simulink model "Full_Car"  (R2020b)
settings: Solver=ode23  StartTime=0.0  StopTime=20  RelTol=0.01  AbsTol=0.01
PreLoadFcn:
  | addpath('Functions');
  | addpath('Functions/geometric');
  | addpath('Parameters');
  | vhclParams;
  | Mass = Sprung_mass + Driver_mass + Unsprung_mass_front*2 + Unsprung_mass_rear*2;
  | COG = (Sprung_mass_COG*Sprung_mass + Driver_COG*Driver_mass + Unsprung_mass_COG_front*Unsprung_mass_front*2.*[1 0 1] + Unsprung_mass_COG_rear*Unsprung_mass_rear*2.*[1 0 1])/Mass;
InitFcn:
  | [folder, ~, ~] = fileparts(which (bdroot));
  | cd(folder);
undefined symbols (not defined in the file): aero_CF, aero_CM, aero_pressureCenter, aero_refArea, airDensity, ARB_bcrnk_frontL, ARB_bcrnk_frontR, ARB_bcrnk_rearL, ARB_bcrnk_rearR, ARB_center_front, ARB_center_rear, ARB_damping_front, ARB_damping_rear, ARB_length_front, ARB_length_rear, ARB_pinSlot_correctionFactor_front, ARB_pinSlot_correctionFactor_rear, ARB_stiffness_front, ARB_stiffness_rear, ARB_TieRod_length_front, ARB_TieRod_length_rear, Damper_coefficient_front, Damper_coefficient_rear, Driver_COG, Driver_mass, DriveTrain_brkwyTrq, DriveTrain_brkwyVel, DriveTrain_CoulombTrq, DriveTrain_effcy, DriveTrain_ratio, DriveTrain_viscCoef, Floor_pos, Floor_size, FS_camber, FS_toe, gravity, Grid_size, initialPosition, Rim_mass, RodDiameter, RodThickness, RS_camber, RS_toe, sigbuilder_block, Spring_preload_front, Spring_preload_rear, Spring_stiffness_front, Spring_stiffness_rear, Sprung_mass, Sprung_mass_COG, Tire_mass, Unsprung_mass_COG_front, Unsprung_mass_COG_rear, Unsprung_mass_front, Unsprung_mass_rear, util_generate_grid_x, util_generate_grid_y, util_rodCrossSection, X, X_F ... (+3)
libraries: sm_lib x385, nesl_utility x79, simulink_extras x8, tire x4, fl_ps_legacy_lib x1
(part 2 of 17)

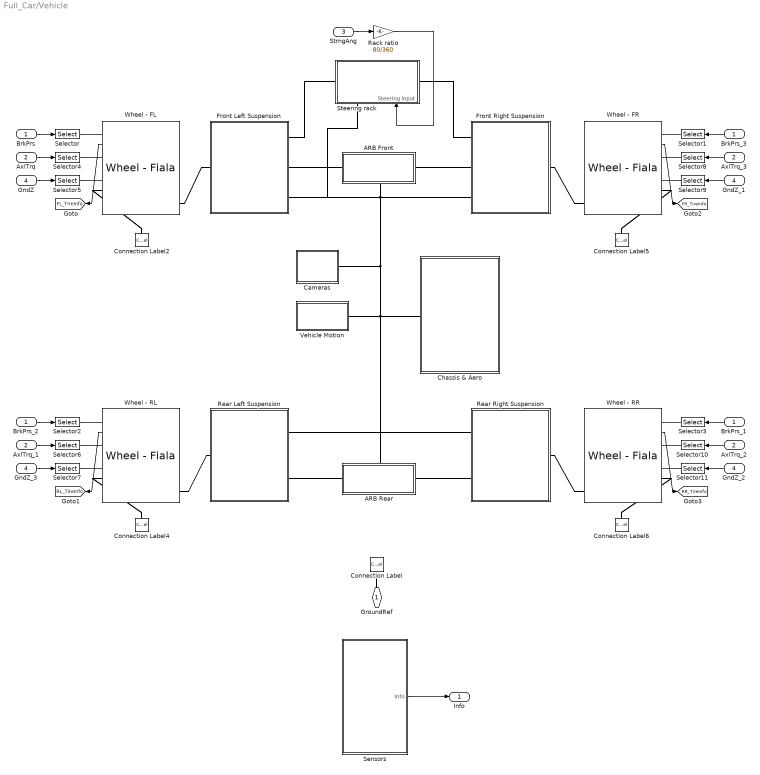
== system Full_Car/Vehicle ==
  BrkPrs : Inport
  AxlTrq : Inport  Port=2
  StrngAng : Inport  Port=3
  GndZ : Inport  Port=4
  ARB Front : Subsystem  mask{arbStiffness=ARB_stiffness_front, arbDamp=ARB_damping_front, arbCorrection=ARB_pinSlot_correctionFactor_front, arbCenter=ARB_center_front, arbLength=ARB_length_front, arbRodLength=ARB_TieRod_length_front, RodDiam=RodDiameter, RodThick=RodThickness}
      mask prompts: arbStiffness "Anti-roll Bar Stiffness"; arbDamp "Anti-roll Bar Damping"; arbCorrection "Stiffness correction factor"; arbCenter "Anti-roll Bar center"; arbLength "Anti-roll Bar length"; arbRodLength "Anti-roll Bar tie-rod length"; RodDiam "Rod diameter"; RodThick "Rod thickness"
      settings: PortSchema="{\"entries\":[{\"content\":{\"sides\":[{\"content\":{\"connectorIds\":[\"LConn1\"]},\"type\":\"ConnectorPlacement.EquallySpacedRectSide\",\"uuid\":\"7b39e7e2-1c99-4477-9d88-8155f0dcbcfa\"},{\"content\":{\"connectorIds\":[\"RConn1\"],\"side\":\"RIGHT\"},\"type\":\"ConnectorPlacement.EquallySpacedRectSide\",\"uuid\":\"e62feb70-34ca-4fb6-b792-0423ade04344\"},{\"content\":{\"connectorIds\":[],\"side\":\"TOP\"},\"type\":\"ConnectorPlacement.EquallySpacedRectSide\",\"uuid\":\"b26fb64d-892c-44a6-8f06-11f439d11cb6\"},{\"content\":{\"connectorIds\":[\"RConn2\"],\"side\":\"BOTTOM\"},\"type\":\"ConnectorPlacement.EquallySpacedRectSide\",\"uuid\":\"e4489596-2281-46d7-9d2e-4ef3bf65c061\"}]},\"type\":\"ConnectorPlacement.EquallySpacedConnector\",\"uuid\":\"4e7c908e-7a44-4075-b019-e55e6c824031\"}],\"packageUris\":[\"http://schema.mathworks.com/mf0/Connector/19700101\"],\"version\":\"1.0\"}"
      mask DialogControl DescGroupVar Prompt: Anti-roll Bar
      mask DialogControl DescTextVar Prompt: Z-Type anti-roll bar assembly
      mask DialogControl ParameterGroupVar Prompt: Dynamics
      mask DialogControl arbStiffness Tooltip: Anti-roll bar stiffness (N/mm)
      mask DialogControl arbDamp Tooltip: Anti-roll bar damping (N/(mm/s))
      mask DialogControl Container4 Prompt: Geometry
      mask DialogControl arbCenter Tooltip: Coordinates of the anti-roll bar center (mm)
      mask DialogControl arbLength Tooltip: Length of the anti-roll bar arm (mm)
      mask DialogControl arbRodLength Tooltip: length of the actuation rod of the anti-roll bar (mm)
      mask DialogControl Container5 Prompt: Graphic
      mask Display: image('$imagefile'); % Use "Edit Image" on toolstrip to change.
  ARB Rear : Subsystem  mask{arbStiffness=ARB_stiffness_rear, arbDamp=ARB_damping_rear, arbCorrection=ARB_pinSlot_correctionFactor_rear, arbCenter=ARB_center_rear, arbLength=ARB_length_rear, arbRodLength=ARB_TieRod_length_rear, RodDiam=RodDiameter, RodThick=RodThickness}
      mask prompts: arbStiffness "Anti-roll Bar Stiffness"; arbDamp "Anti-roll Bar Damping"; arbCorrection "Stiffness correction factor"; arbCenter "Anti-roll Bar center"; arbLength "Anti-roll Bar length"; arbRodLength "Anti-roll Bar tie-rod length"; RodDiam "Rod diameter"; RodThick "Rod thickness"
      settings: PortSchema="{\"entries\":[{\"content\":{\"sides\":[{\"content\":{\"connectorIds\":[\"LConn1\"]},\"type\":\"ConnectorPlacement.EquallySpacedRectSide\",\"uuid\":\"7b39e7e2-1c99-4477-9d88-8155f0dcbcfa\"},{\"content\":{\"connectorIds\":[\"RConn1\"],\"side\":\"RIGHT\"},\"type\":\"ConnectorPlacement.EquallySpacedRectSide\",\"uuid\":\"e62feb70-34ca-4fb6-b792-0423ade04344\"},{\"content\":{\"connectorIds\":[],\"side\":\"TOP\"},\"type\":\"ConnectorPlacement.EquallySpacedRectSide\",\"uuid\":\"b26fb64d-892c-44a6-8f06-11f439d11cb6\"},{\"content\":{\"connectorIds\":[\"RConn2\"],\"side\":\"BOTTOM\"},\"type\":\"ConnectorPlacement.EquallySpacedRectSide\",\"uuid\":\"e4489596-2281-46d7-9d2e-4ef3bf65c061\"}]},\"type\":\"ConnectorPlacement.EquallySpacedConnector\",\"uuid\":\"4e7c908e-7a44-4075-b019-e55e6c824031\"}],\"packageUris\":[\"http://schema.mathworks.com/mf0/Connector/19700101\"],\"version\":\"1.0\"}"
      mask DialogControl DescGroupVar Prompt: Anti-roll Bar
      mask DialogControl DescTextVar Prompt: Z-Type anti-roll bar assembly
      mask DialogControl ParameterGroupVar Prompt: Dynamics
      mask DialogControl arbStiffness Tooltip: Anti-roll bar stiffness (N/mm)
      mask DialogControl arbDamp Tooltip: Anti-roll bar damping (N/(mm/s))
      mask DialogControl Container4 Prompt: Geometry
      mask DialogControl arbCenter Tooltip: Coordinates of the anti-roll bar center (mm)
      mask DialogControl arbLength Tooltip: Length of the anti-roll bar arm (mm)
      mask DialogControl arbRodLength Tooltip: length of the actuation rod of the anti-roll bar (mm)
      mask DialogControl Container5 Prompt: Graphic
      mask Display: image('$imagefile'); % Use "Edit Image" on toolstrip to change.
  AxlTrq_1 : InportShadow  Port=2
  AxlTrq_2 : InportShadow  Port=2
  AxlTrq_3 : InportShadow  Port=2
  BrkPrs_1 : InportShadow
  BrkPrs_2 : InportShadow
  BrkPrs_3 : InportShadow
  Cameras : Subsystem
      mask Display: image('$imagefile'); % Use "Edit Image" on toolstrip to change.
  Chassis & Aero : Subsystem
      settings: PortSchema="{\"entries\":[{\"content\":{\"sides\":[{\"content\":{\"connectorIds\":[]},\"type\":\"ConnectorPlacement.EquallySpacedRectSide\",\"uuid\":\"cbbe6af3-19fb-48b3-8800-b215223b12f6\"},{\"content\":{\"connectorIds\":[],\"side\":\"RIGHT\"},\"type\":\"ConnectorPlacement.EquallySpacedRectSide\",\"uuid\":\"e0ce8cb6-c1e3-4be9-8f24-6f1308fe5f38\"},{\"content\":{\"connectorIds\":[\"LConn1\"],\"side\":\"TOP\"},\"type\":\"ConnectorPlacement.EquallySpacedRectSide\",\"uuid\":\"4e525be5-d309-4877-ab04-513b663981dd\"},{\"content\":{\"connectorIds\":[],\"side\":\"BOTTOM\"},\"type\":\"ConnectorPlacement.EquallySpacedRectSide\",\"uuid\":\"1a8d8adb-4793-4ada-a6b7-ecb65b96140c\"}]},\"type\":\"ConnectorPlacement.EquallySpacedConnector\",\"uuid\":\"0639ed7d-0262-43c4-ade7-52e76f974124\"}],\"packageUris\":[\"http://schema.mathworks.com/mf0/Connector/19700101\"],\"version\":\"1.0\"}"
      mask Display:
      | image('$imagefile'); % Use "Edit Image" on toolstrip to change.
      | port_label('LConn',1,'Ref')
  Connection Label{,2,4..6} (5 alike) : ConnectionLabel  Label=GroundRef
  Front Left Suspension : Subsystem  mask{X=X_F, side=Left, sideSign=1, ARB_bcrnk=ARB_bcrnk_frontL, Camber=FS_camber, Toe=FS_toe, BcrnkPos=0, DamperPos=[0 90 0], ARB_On=on, St_On=on, SpringStiffness=Spring_stiffness_front, SpringPreload=Spring_preload_front, DamperCoef=Damper_coefficient_front, DamperMaxExt=200, DampLimit_MaxK=10000, DampLimit_MaxC=1, DampLimit_MaxTrans=1, DamperMinExt=154, DampLimit_MinK=2000, DampLimit_MinC=1, DampLimit_MinTrans=5, PushSetupLength=0, ToeSetupLength=0, UnsprungMass=Unsprung_mass_front-Tire_mass-Rim_mass, UnsprungMassCOG=Unsprung_mass_COG_front, RodThick=1, RodDiam=14, UCAfore_X=152.984798840791, UCAaft_X=-127.305532779834, UCA_Y=-257.3812, UCA_pushBracket_X=1.13049065291656, UCA_pushBracket_Y=-16.0659716079757, UCA_pushBracket_Z=19.373063236757, LCAfore_X=67.6445283762746, LCAaft_X=-143.178288225724, LCA_Y=-328.86366138683, UCA_Rot=[0.0129952164868874 0.0511310087922121 0.998607402480249;0.0511310087922121 0.997351198186899 -0.0517320733689305;-0.998607402480249 0.0517320733689305 0.0103464146737861], LCA_Rot=[0.000690445618848567 0.0146957287408994 0.99989177356433;0.0146957287408994 0.999783886342046 -0.0147042907877108;-0.99989177356433 0.0147042907877108 0.000474331960894192], BcrnkDamp=69.0006325768104, BcrnkPush=69.0007956041088, BcrnkAng=54.9995482296285, BcrnkRot=[-0.0310893815864683 0.0519417534127774 0.998166070654066;0.0519417534127774 0.997383402646003 -0.0502832216418927;-0.998166070654066 0.0502832216418927 -0.0337059789404655]}
      mask prompts: X "Coordinates"; ARB_bcrnk "ARB tie-rod"; BcrnkPos "Bellcrank initial rotation"; DamperPos "Damper initial position"; ARB_On "Anti-roll bar"; St_On "Steering"; SpringStiffness "Spring stiffness"; SpringPreload "Spring preload"; DamperCoef "Damping Coefficient"; DamperMaxExt "Maximum extension"; DampLimit_MaxK "Max_Stiffness"; DampLimit_MaxC "Max_Damping"; DampLimit_MaxTrans "Max_Transition"; DamperMinExt "Maximum compression"; DampLimit_MinK "Min_Stiffness"; DampLimit_MinC "Min_Damping"; DampLimit_MinTrans "Min_Transition"; PushSetupLength "Push"; ToeSetupLength "Toe"; UnsprungMass "Mass"; UnsprungMassCOG "COG"; RodThick "Rod Thickness"; RodDiam "Rod Diameter"
      mask help: Define a Simscape Multibody model of a double control arm independent suspension
      mask init:
      | dblAarmSusp_init(gcb)
      mask Help:
      | -Geometry:
      |     +Suspension Coordinates: coordinates of the points that define a double wishbone suspension system. The coordinates must always correspond to the LEFT side. The variable (e.g. 'X') containing the data must be a row vector with the following values, in order:
      |       1-A-Arm Upper Front hardpoint. X([1 2 3])
      |       2-A-Arm Upper Rear. X([4 5 6])
      |       3-A-Arm Lower Front. X([7 8 9])
      |       4-A-Arm Lower Rear. X([10 11 12])
      |       5-A-Arm Upper Upright. X([13 14 15])
      |       6-A-Arm Lower Upright. X([16 17 18])
      |       7-Steering Rack. X([19 20 21])
      |       8-Steering Upright. X([22 23 24])
      |       9-Wheel Center. X([25 26 27])
      |       10-Wheel Plane normal vector. X([28 29 30])
      |       11-A-Arm Push Bracket. X([31 32 33])
      |       12-Bellcrank Push Bracket. X([34 35 36])
      |       13-Bellcrank Chassis Bracket. X([37 38 39])
      |       14-Bellcrank Damper Bracket. X([40 41 42])
      |       15-Damper Bracket. X([43 44 45])
      |       16-Bellcrank ARB Bracket. X(46:48)
      |       17-ARB end. X(49:51)
      |       18-ARB center. X(52:54)
      |     +It must be noted that in the model the bellcranks have the same dimensions as defined in the geometry, but the position and orientation is diferent. It is positioned in a vertical plane that contains the a-arm push bracket. The reason is to let the anti-roll bar be symmetric.
      mask TypeOptions side: Left | Right
      mask Callback side:
      | switch get_param(gcb,'side')
      |     case 'Left'
      |         set_param(gcb,'sideSign','1');
      |     case 'Right'
      |         set_param(gcb,'sideSign','-1');
      | end
      mask DialogControl DescGroupVar Prompt: Double Wishbone Suspension
      mask DialogControl SuspGeomTab Prompt: Geometry
      mask DialogControl X Tooltip: Variable containting suspension points coordinates
      mask DialogControl Container22 Prompt: Anti-roll Bar
      mask DialogControl ARB_bcrnk Tooltip: Coordinates of the attachment point of the ARB to the bellcrank (mm)
      mask DialogControl Container8 Prompt: Wheel angles
      mask DialogControl Camber Tooltip: Camber angle in deg at reference ride height
      mask DialogControl Toe Tooltip: Toe angle in deg at reference ride height
      mask DialogControl Container23 Prompt: Assembly
      mask DialogControl BcrnkPos Tooltip: Degrees of rotation of follower frame respect to base frame of the bellcrank bracket joint for the inital position
      mask DialogControl DamperPos Tooltip: Degrees of rotation of follower frame respect to base frame of the bellcrank bracket joint for the inital position, specified as a rotation sequence X-Y-Z
      mask DialogControl ARB_On Tooltip: Select wheter the block displays connection for ARB or not
      mask DialogControl St_On Tooltip: Select wether the block displays steering input or not
      mask DialogControl SpringDampTab Prompt: Spring and Damper
      mask DialogControl SpringStiffness Tooltip: Spring stiffness (lb/inch)
      mask DialogControl SpringPreload Tooltip: Spring preload in mm
      mask DialogControl DamperCoef Tooltip: Damping Coefficient in N/(mm/s)
      mask DialogControl Container9 Prompt: Limits
      mask DialogControl DamperMaxExt Tooltip: Maximum allowable extension of the damper, meassured between ball-joints. This value is also used for the natural length of the spring (mm)
      mask DialogControl DampLimit_MaxK Tooltip: Stiffness when upper limit is reached in N/mm
      mask DialogControl DampLimit_MaxC Tooltip: Damping coefficient when upper limit is reached in N/(mm/s)
      mask DialogControl DampLimit_MaxTrans Tooltip: Transition region width between normal behavior and limit
      mask DialogControl DamperMinExt Tooltip: Minimum allowable extension (max compression) of the damper, meassured between ball-joints (mm)
      mask DialogControl DampLimit_MinK Tooltip: Stiffness when lower limit is reached in N/(mm/s)
      mask DialogControl DampLimit_MinC Tooltip: Damping coefficient when lower limit is reached in N/(mm/s)
      mask DialogControl DampLimit_MinTrans Tooltip: Transition region width between normal behavior and limit
      mask DialogControl Container13 Prompt: Setup
      mask DialogControl PushSetupLength Tooltip: Length adjustment of the rod ends in the push rod
      mask DialogControl ToeSetupLength Tooltip: Length adjustment of the rod ends in the toe/steering rod
      mask DialogControl Container19 Prompt: Mass
      mask DialogControl Container20 Prompt: Non-rotating unsprung mass
      mask DialogControl UnsprungMass Tooltip: Non rotating unsprung mass (kg)
      mask DialogControl UnsprungMassCOG Tooltip: Non-rotating unsprung mass center of gravity coordinates (mm)
      mask DialogControl Container17 Prompt: Graphic
      mask DialogControl internalParamContainer Prompt: Internal params
      mask Display: image('$imagefile'); % Use "Edit Image" on toolstrip to change.
  Front Right Suspension : Subsystem  mask{X=X_F, side=Right, sideSign=-1, ARB_bcrnk=ARB_bcrnk_frontR, Camber=FS_camber, Toe=FS_toe, BcrnkPos=0, DamperPos=[0 -90 180], ARB_On=on, St_On=on, SpringStiffness=Spring_stiffness_front, SpringPreload=Spring_preload_front, DamperCoef=Damper_coefficient_front, DamperMaxExt=200, DampLimit_MaxK=10000, DampLimit_MaxC=1, DampLimit_MaxTrans=1, DamperMinExt=154, DampLimit_MinK=2000, DampLimit_MinC=1, DampLimit_MinTrans=5, PushSetupLength=0, ToeSetupLength=0, UnsprungMass=Unsprung_mass_front-Tire_mass-Rim_mass, UnsprungMassCOG=Unsprung_mass_COG_front, RodThick=1, RodDiam=14, UCAfore_X=152.984798840791, UCAaft_X=-127.305532779834, UCA_Y=-257.3812, UCA_pushBracket_X=1.13049065291656, UCA_pushBracket_Y=-16.0659716079757, UCA_pushBracket_Z=19.373063236757, LCAfore_X=67.6445283762746, LCAaft_X=-143.178288225724, LCA_Y=-328.86366138683, UCA_Rot=[0.0129952164868874 -0.0511310087922121 0.998607402480249;-0.0511310087922121 0.997351198186899 0.0517320733689305;-0.998607402480249 -0.0517320733689305 0.0103464146737861], LCA_Rot=[0.000690445618848567 -0.0146957287408994 0.99989177356433;-0.0146957287408994 0.999783886342046 0.0147042907877108;-0.99989177356433 -0.0147042907877108 0.000474331960894192], BcrnkDamp=69.0006325768104, BcrnkPush=69.0007956041088, BcrnkAng=54.9995482296285, BcrnkRot=[-0.0310893815864683 -0.0519417534127774 0.998166070654066;-0.0519417534127774 0.997383402646003 0.0502832216418927;-0.998166070654066 -0.0502832216418927 -0.0337059789404655]}
      mask prompts: X "Coordinates"; ARB_bcrnk "ARB tie-rod"; BcrnkPos "Bellcrank initial rotation"; DamperPos "Damper initial position"; ARB_On "Anti-roll bar"; St_On "Steering"; SpringStiffness "Spring stiffness"; SpringPreload "Spring preload"; DamperCoef "Damping Coefficient"; DamperMaxExt "Maximum extension"; DampLimit_MaxK "Max_Stiffness"; DampLimit_MaxC "Max_Damping"; DampLimit_MaxTrans "Max_Transition"; DamperMinExt "Maximum compression"; DampLimit_MinK "Min_Stiffness"; DampLimit_MinC "Min_Damping"; DampLimit_MinTrans "Min_Transition"; PushSetupLength "Push"; ToeSetupLength "Toe"; UnsprungMass "Mass"; UnsprungMassCOG "COG"; RodThick "Rod Thickness"; RodDiam "Rod Diameter"
      mask help: Define a Simscape Multibody model of a double control arm independent suspension
      mask init:
      | dblAarmSusp_init(gcb)
      mask Help:
      | -Geometry:
      |     +Suspension Coordinates: coordinates of the points that define a double wishbone suspension system. The coordinates must always correspond to the LEFT side. The variable (e.g. 'X') containing the data must be a row vector with the following values, in order:
      |       1-A-Arm Upper Front hardpoint. X([1 2 3])
      |       2-A-Arm Upper Rear. X([4 5 6])
      |       3-A-Arm Lower Front. X([7 8 9])
      |       4-A-Arm Lower Rear. X([10 11 12])
      |       5-A-Arm Upper Upright. X([13 14 15])
      |       6-A-Arm Lower Upright. X([16 17 18])
      |       7-Steering Rack. X([19 20 21])
      |       8-Steering Upright. X([22 23 24])
      |       9-Wheel Center. X([25 26 27])
      |       10-Wheel Plane normal vector. X([28 29 30])
      |       11-A-Arm Push Bracket. X([31 32 33])
      |       12-Bellcrank Push Bracket. X([34 35 36])
      |       13-Bellcrank Chassis Bracket. X([37 38 39])
      |       14-Bellcrank Damper Bracket. X([40 41 42])
      |       15-Damper Bracket. X([43 44 45])
      |       16-Bellcrank ARB Bracket. X(46:48)
      |       17-ARB end. X(49:51)
      |       18-ARB center. X(52:54)
      |     +It must be noted that in the model the bellcranks have the same dimensions as defined in the geometry, but the position and orientation is diferent. It is positioned in a vertical plane that contains the a-arm push bracket. The reason is to let the anti-roll bar be symmetric.
      mask TypeOptions side: Left | Right
      mask Callback side:
      | switch get_param(gcb,'side')
      |     case 'Left'
      |         set_param(gcb,'sideSign','1');
      |     case 'Right'
      |         set_param(gcb,'sideSign','-1');
      | end
      mask DialogControl DescGroupVar Prompt: Double Wishbone Suspension
      mask DialogControl SuspGeomTab Prompt: Geometry
      mask DialogControl X Tooltip: Variable containting suspension points coordinates
      mask DialogControl Container22 Prompt: Anti-roll Bar
      mask DialogControl ARB_bcrnk Tooltip: Coordinates of the attachment point of the ARB to the bellcrank (mm)
      mask DialogControl Container8 Prompt: Wheel angles
      mask DialogControl Camber Tooltip: Camber angle in deg at reference ride height
      mask DialogControl Toe Tooltip: Toe angle in deg at reference ride height
      mask DialogControl Container23 Prompt: Assembly
      mask DialogControl BcrnkPos Tooltip: Degrees of rotation of follower frame respect to base frame of the bellcrank bracket joint for the inital position
      mask DialogControl DamperPos Tooltip: Degrees of rotation of follower frame respect to base frame of the bellcrank bracket joint for the inital position, specified as a rotation sequence X-Y-Z
      mask DialogControl ARB_On Tooltip: Select wheter the block displays connection for ARB or not
      mask DialogControl St_On Tooltip: Select wether the block displays steering input or not
      mask DialogControl SpringDampTab Prompt: Spring and Damper
      mask DialogControl SpringStiffness Tooltip: Spring stiffness in lb/inch
      mask DialogControl SpringPreload Tooltip: Spring preload in mm
      mask DialogControl DamperCoef Tooltip: Damping Coefficient in N/(mm/s)
      mask DialogControl Container9 Prompt: Limits
      mask DialogControl DamperMaxExt Tooltip: Maximum allowable extension of the damper, meassured between ball-joints (mm)
      mask DialogControl DampLimit_MaxK Tooltip: Stiffness when upper limit is reached in N/mm
      mask DialogControl DampLimit_MaxC Tooltip: Damping coefficient when upper limit is reached in N/(mm/s)
      mask DialogControl DampLimit_MaxTrans Tooltip: Transition region width between normal behavior and limit
      mask DialogControl DamperMinExt Tooltip: Minimum allowable extension (max compression) of the damper, meassured between ball-joints (mm)
      mask DialogControl DampLimit_MinK Tooltip: Stiffness when lower limit is reached in N/(mm/s)
      mask DialogControl DampLimit_MinC Tooltip: Damping coefficient when lower limit is reached in N/(mm/s)
      mask DialogControl DampLimit_MinTrans Tooltip: Transition region width between normal behavior and limit
      mask DialogControl Container13 Prompt: Setup
      mask DialogControl PushSetupLength Tooltip: Length adjustment of the rod ends in the push rod
      mask DialogControl ToeSetupLength Tooltip: Length adjustment of the rod ends in the toe/steering rod
      mask DialogControl Container19 Prompt: Mass
      mask DialogControl Container20 Prompt: Non-rotating unsprung mass
      mask DialogControl UnsprungMass Tooltip: Non rotating unsprung mass (kg)
      mask DialogControl UnsprungMassCOG Tooltip: Non-rotating unsprung mass center of gravity coordinates (mm)
      mask DialogControl Container17 Prompt: Graphic
      mask DialogControl internalParamContainer Prompt: Internal params
      mask Display: image('$imagefile'); % Use "Edit Image" on toolstrip to change.
  GndZ_1 : InportShadow  Port=4
  GndZ_2 : InportShadow  Port=4
  GndZ_3 : InportShadow  Port=4
  Rack ratio : Gain  Gain=80/360
  Rear Left Suspension : Subsystem  mask{X=X_R, side=Left, sideSign=1, ARB_bcrnk=ARB_bcrnk_rearL, Camber=RS_camber, Toe=RS_toe, BcrnkPos=0, DamperPos=[0 90 0], ARB_On=on, St_On=on, SpringStiffness=Spring_stiffness_rear, SpringPreload=Spring_preload_rear, DamperCoef=Damper_coefficient_rear, DamperMaxExt=200, DampLimit_MaxK=10000, DampLimit_MaxC=1, DampLimit_MaxTrans=1, DamperMinExt=154, DampLimit_MinK=2000, DampLimit_MinC=1, DampLimit_MinTrans=5, PushSetupLength=0, ToeSetupLength=0, UnsprungMass=Unsprung_mass_rear-Tire_mass-Rim_mass, UnsprungMassCOG=Unsprung_mass_COG_rear, RodThick=1, RodDiam=14, UCAfore_X=112.908254553074, UCAaft_X=-151.189265314115, UCA_Y=-248.704, UCA_pushBracket_X=-1.98922125533095, UCA_pushBracket_Y=-17.1299173592706, UCA_pushBracket_Z=18.3706211670128, LCAfore_X=167.7, LCAaft_X=-138.2, LCA_Y=-328.9, UCA_Rot=[-0.00194921998470532 -0.0910516591545036 0.995844262877817;-0.0910516591545036 0.991725723749838 0.0904968740790107;-0.995844262877817 -0.0904968740790107 -0.0102234962348675], LCA_Rot=[0 0 1;0 1 0;-1 0 0], BcrnkDamp=80.0368909948768, BcrnkPush=67.0449200519613, BcrnkAng=59.9638794672988, BcrnkRot=[0.047890311837444 -0.016573734999306 0.998715089172225;-0.016573734999306 0.999711494699358 0.0173850129176741;-0.998715089172225 -0.0173850129176741 0.0476018065368023]}
      mask prompts: X "Coordinates"; ARB_bcrnk "ARB tie-rod"; BcrnkPos "Bellcrank initial rotation"; DamperPos "Damper initial position"; ARB_On "Anti-roll bar"; St_On "Steering"; SpringStiffness "Spring stiffness"; SpringPreload "Spring preload"; DamperCoef "Damping Coefficient"; DamperMaxExt "Maximum extension"; DampLimit_MaxK "Max_Stiffness"; DampLimit_MaxC "Max_Damping"; DampLimit_MaxTrans "Max_Transition"; DamperMinExt "Maximum compression"; DampLimit_MinK "Min_Stiffness"; DampLimit_MinC "Min_Damping"; DampLimit_MinTrans "Min_Transition"; PushSetupLength "Push"; ToeSetupLength "Toe"; UnsprungMass "Mass"; UnsprungMassCOG "COG"; RodThick "Rod Thickness"; RodDiam "Rod Diameter"
      mask help: Define a Simscape Multibody model of a double control arm independent suspension
      mask init:
      | dblAarmSusp_init(gcb)
      mask Help:
      | -Geometry:
      |     +Suspension Coordinates: coordinates of the points that define a double wishbone suspension system. The coordinates must always correspond to the LEFT side. The variable (e.g. 'X') containing the data must be a row vector with the following values, in order:
      |       1-A-Arm Upper Front hardpoint. X([1 2 3])
      |       2-A-Arm Upper Rear. X([4 5 6])
      |       3-A-Arm Lower Front. X([7 8 9])
      |       4-A-Arm Lower Rear. X([10 11 12])
      |       5-A-Arm Upper Upright. X([13 14 15])
      |       6-A-Arm Lower Upright. X([16 17 18])
      |       7-Steering Rack. X([19 20 21])
      |       8-Steering Upright. X([22 23 24])
      |       9-Wheel Center. X([25 26 27])
      |       10-Wheel Plane normal vector. X([28 29 30])
      |       11-A-Arm Push Bracket. X([31 32 33])
      |       12-Bellcrank Push Bracket. X([34 35 36])
      |       13-Bellcrank Chassis Bracket. X([37 38 39])
      |       14-Bellcrank Damper Bracket. X([40 41 42])
      |       15-Damper Bracket. X([43 44 45])
      |       16-Bellcrank ARB Bracket. X(46:48)
      |       17-ARB end. X(49:51)
      |       18-ARB center. X(52:54)
      |     +It must be noted that in the model the bellcranks have the same dimensions as defined in the geometry, but the position and orientation is diferent. It is positioned in a vertical plane that contains the a-arm push bracket. The reason is to let the anti-roll bar be symmetric.
      | 
      | -Graphic: a CAD file can be specified to graphically represent some parts of the suspension. In order for the part to appear in its proper position, its reference axis and origin in the CAD file must be the same as the reference for the suspension points coordinates.
      mask TypeOptions side: Left | Right
      mask Callback side:
      | switch get_param(gcb,'side')
      |     case 'Left'
      |         set_param(gcb,'sideSign','1');
      |     case 'Right'
      |         set_param(gcb,'sideSign','-1');
      | end
      mask DialogControl DescGroupVar Prompt: Double Wishbone Suspension
      mask DialogControl SuspGeomTab Prompt: Geometry
      mask DialogControl X Tooltip: Variable containting suspension points coordinates
      mask DialogControl Container23 Prompt: Anti-roll bar
      mask DialogControl ARB_bcrnk Tooltip: Coordinates of the attachment point of the anti-roll bar to the bellcrank (mm)
      mask DialogControl Container8 Prompt: Wheel angles
      mask DialogControl Camber Tooltip: Camber angle in deg at reference ride height
      mask DialogControl Toe Tooltip: Toe angle in deg at reference ride height
      mask DialogControl Container22 Prompt: Assembly
      mask DialogControl BcrnkPos Tooltip: Degrees of rotation of follower frame respect to base frame of the bellcrank bracket joint for the inital position
      mask DialogControl DamperPos Tooltip: Degrees of rotation of follower frame respect to base frame of the bellcrank bracket joint for the inital position, specified as a rotation sequence X-Y-Z
      mask DialogControl ARB_On Tooltip: Select wheter the block displays connection for ARB or not
      mask DialogControl St_On Tooltip: Select wether the block displays steering input or not
      mask DialogControl SpringDampTab Prompt: Spring and Damper
      mask DialogControl SpringStiffness Tooltip: Spring stiffness in lb/inch
      mask DialogControl SpringPreload Tooltip: Spring preload in mm
      mask DialogControl DamperCoef Tooltip: Damping Coefficient in N/(mm/s)
      mask DialogControl Container9 Prompt: Limits
      mask DialogControl DamperMaxExt Tooltip: Maximum allowable extension of the damper, meassured between ball-joints (mm)
      mask DialogControl DampLimit_MaxK Tooltip: Stiffness when upper limit is reached in N/mm
      mask DialogControl DampLimit_MaxC Tooltip: Damping coefficient when upper limit is reached in N/(mm/s)
      mask DialogControl DampLimit_MaxTrans Tooltip: Transition region width between normal behavior and limit
      mask DialogControl DamperMinExt Tooltip: Minimum allowable extension (max compression) of the damper, meassured between ball-joints (mm)
      mask DialogControl DampLimit_MinK Tooltip: Stiffness when lower limit is reached in N/(mm/s)
      mask DialogControl DampLimit_MinC Tooltip: Damping coefficient when lower limit is reached in N/(mm/s)
      mask DialogControl DampLimit_MinTrans Tooltip: Transition region width between normal behavior and limit
      mask DialogControl Container13 Prompt: Setup
      mask DialogControl PushSetupLength Tooltip: Length adjustment of the rod ends in the push rod
      mask DialogControl ToeSetupLength Tooltip: Length adjustment of the rod ends in the toe/steering rod
      mask DialogControl Container19 Prompt: Mass
      mask DialogControl Container20 Prompt: Non-rotating unsprung mass
      mask DialogControl UnsprungMass Tooltip: Non rotating unsprung mass (kg)
      mask DialogControl UnsprungMassCOG Tooltip: Non-rotating unsprung mass center of gravity coordinates (mm)
      mask DialogControl Container17 Prompt: Graphic
      mask DialogControl internalParamContainer Prompt: Internal params
      mask Display: image('$imagefile'); % Use "Edit Image" on toolstrip to change.
  Rear Right Suspension : Subsystem  mask{X=X_R, side=Right, sideSign=-1, ARB_bcrnk=ARB_bcrnk_rearR, Camber=RS_camber, Toe=RS_toe, BcrnkPos=0, DamperPos=[0 90 0], ARB_On=on, St_On=on, SpringStiffness=Spring_stiffness_rear, SpringPreload=Spring_preload_rear, DamperCoef=Damper_coefficient_rear, DamperMaxExt=200, DampLimit_MaxK=10000, DampLimit_MaxC=1, DampLimit_MaxTrans=1, DamperMinExt=154, DampLimit_MinK=2000, DampLimit_MinC=1, DampLimit_MinTrans=5, PushSetupLength=0, ToeSetupLength=0, UnsprungMass=Unsprung_mass_rear-Tire_mass-Rim_mass, UnsprungMassCOG=Unsprung_mass_COG_rear, RodThick=1, RodDiam=14, UCAfore_X=112.908254553074, UCAaft_X=-151.189265314115, UCA_Y=-248.704, UCA_pushBracket_X=-1.98922125533095, UCA_pushBracket_Y=-17.1299173592706, UCA_pushBracket_Z=18.3706211670128, LCAfore_X=167.7, LCAaft_X=-138.2, LCA_Y=-328.9, UCA_Rot=[-0.00194921998470532 0.0910516591545036 0.995844262877817;0.0910516591545036 0.991725723749838 -0.0904968740790107;-0.995844262877817 0.0904968740790107 -0.0102234962348675], LCA_Rot=[0 0 1;0 1 0;-1 0 0], BcrnkDamp=80.0368909948768, BcrnkPush=67.0449200519613, BcrnkAng=59.9638794672988, BcrnkRot=[0.047890311837444 0.016573734999306 0.998715089172225;0.016573734999306 0.999711494699358 -0.0173850129176741;-0.998715089172225 0.0173850129176741 0.0476018065368023]}
      mask prompts: X "Coordinates"; ARB_bcrnk "ARB tie-rod"; BcrnkPos "Bellcrank initial rotation"; DamperPos "Damper initial position"; ARB_On "Anti-roll bar"; St_On "Steering"; SpringStiffness "Spring stiffness"; SpringPreload "Spring preload"; DamperCoef "Damping Coefficient"; DamperMaxExt "Maximum extension"; DampLimit_MaxK "Max_Stiffness"; DampLimit_MaxC "Max_Damping"; DampLimit_MaxTrans "Max_Transition"; DamperMinExt "Maximum compression"; DampLimit_MinK "Min_Stiffness"; DampLimit_MinC "Min_Damping"; DampLimit_MinTrans "Min_Transition"; PushSetupLength "Push"; ToeSetupLength "Toe"; UnsprungMass "Mass"; UnsprungMassCOG "COG"; RodThick "Rod Thickness"; RodDiam "Rod Diameter"
      mask help: Define a Simscape Multibody model of a double control arm independent suspension
      mask init:
      | dblAarmSusp_init(gcb)
      mask Help:
      | -Geometry:
      |     +Suspension Coordinates: coordinates of the points that define a double wishbone suspension system. The coordinates must always correspond to the LEFT side. The variable (e.g. 'X') containing the data must be a row vector with the following values, in order:
      |       1-A-Arm Upper Front hardpoint. X([1 2 3])
      |       2-A-Arm Upper Rear. X([4 5 6])
      |       3-A-Arm Lower Front. X([7 8 9])
      |       4-A-Arm Lower Rear. X([10 11 12])
      |       5-A-Arm Upper Upright. X([13 14 15])
      |       6-A-Arm Lower Upright. X([16 17 18])
      |       7-Steering Rack. X([19 20 21])
      |       8-Steering Upright. X([22 23 24])
      |       9-Wheel Center. X([25 26 27])
      |       10-Wheel Plane normal vector. X([28 29 30])
      |       11-A-Arm Push Bracket. X([31 32 33])
      |       12-Bellcrank Push Bracket. X([34 35 36])
      |       13-Bellcrank Chassis Bracket. X([37 38 39])
      |       14-Bellcrank Damper Bracket. X([40 41 42])
      |       15-Damper Bracket. X([43 44 45])
      |       16-Bellcrank ARB Bracket. X(46:48)
      |       17-ARB end. X(49:51)
      |       18-ARB center. X(52:54)
      |     +It must be noted that in the model the bellcranks have the same dimensions as defined in the geometry, but the position and orientation is diferent. It is positioned in a vertical plane that contains the a-arm push bracket. The reason is to let the anti-roll bar be symmetric.
      | 
      | -Graphic: a CAD file can be specified to graphically represent some parts of the suspension. In order for the part to appear in its proper position, its reference axis and origin in the CAD file must be the same as the reference for the suspension points coordinates.
      mask TypeOptions side: Left | Right
      mask Callback side:
      | switch get_param(gcb,'side')
      |     case 'Left'
      |         set_param(gcb,'sideSign','1');
      |     case 'Right'
      |         set_param(gcb,'sideSign','-1');
      | end
      mask DialogControl DescGroupVar Prompt: Double Wishbone Suspension
      mask DialogControl SuspGeomTab Prompt: Geometry
      mask DialogControl X Tooltip: Variable containting suspension points coordinates
      mask DialogControl Container23 Prompt: Anti-roll bar
      mask DialogControl ARB_bcrnk Tooltip: Coordinates of the attachment point of the anti-roll bar to the bellcrank (mm)
      mask DialogControl Container8 Prompt: Wheel angles
      mask DialogControl Camber Tooltip: Camber angle in deg at reference ride height
      mask DialogControl Toe Tooltip: Toe angle in deg at reference ride height
      mask DialogControl Container22 Prompt: Assembly
      mask DialogControl BcrnkPos Tooltip: Degrees of rotation of follower frame respect to base frame of the bellcrank bracket joint for the inital position
      mask DialogControl DamperPos Tooltip: Degrees of rotation of follower frame respect to base frame of the bellcrank bracket joint for the inital position, specified as a rotation sequence X-Y-Z
      mask DialogControl ARB_On Tooltip: Select wheter the block displays connection for ARB or not
      mask DialogControl St_On Tooltip: Select wether the block displays steering input or not
      mask DialogControl SpringDampTab Prompt: Spring and Damper
      mask DialogControl SpringStiffness Tooltip: Spring stiffness in lb/inch
      mask DialogControl SpringPreload Tooltip: Spring preload in mm
      mask DialogControl DamperCoef Tooltip: Damping Coefficient in N/(mm/s)
      mask DialogControl Container9 Prompt: Limits
      mask DialogControl DamperMaxExt Tooltip: Maximum allowable extension of the damper, meassured between ball-joints (mm)
      mask DialogControl DampLimit_MaxK Tooltip: Stiffness when upper limit is reached in N/mm
      mask DialogControl DampLimit_MaxC Tooltip: Damping coefficient when upper limit is reached in N/(mm/s)
      mask DialogControl DampLimit_MaxTrans Tooltip: Transition region width between normal behavior and limit
      mask DialogControl DamperMinExt Tooltip: Minimum allowable extension (max compression) of the damper, meassured between ball-joints (mm)
      mask DialogControl DampLimit_MinK Tooltip: Stiffness when lower limit is reached in N/(mm/s)
      mask DialogControl DampLimit_MinC Tooltip: Damping coefficient when lower limit is reached in N/(mm/s)
      mask DialogControl DampLimit_MinTrans Tooltip: Transition region width between normal behavior and limit
      mask DialogControl Container13 Prompt: Setup
      mask DialogControl PushSetupLength Tooltip: Length adjustment of the rod ends in the push rod
      mask DialogControl ToeSetupLength Tooltip: Length adjustment of the rod ends in the toe/steering rod
      mask DialogControl Container19 Prompt: Mass
      mask DialogControl Container20 Prompt: Non-rotating unsprung mass
      mask DialogControl UnsprungMass Tooltip: Non rotating unsprung mass (kg)
      mask DialogControl UnsprungMassCOG Tooltip: Non-rotating unsprung mass center of gravity coordinates (mm)
      mask DialogControl Container17 Prompt: Graphic
      mask DialogControl internalParamContainer Prompt: Internal params
      mask Display: image('$imagefile'); % Use "Edit Image" on toolstrip to change.
  Selector : Selector  InputPortWidth=4  IndexOptions=Index vector (dialog)  Indices=1  OutputSizes=1
  Selector1 : Selector  InputPortWidth=4  IndexOptions=Index vector (dialog)  Indices=2  OutputSizes=1
  Selector10 : Selector  InputPortWidth=4  IndexOptions=Index vector (dialog)  Indices=4  OutputSizes=1
  Selector11 : Selector  InputPortWidth=4  IndexOptions=Index vector (dialog)  Indices=4  OutputSizes=1
  Selector2 : Selector  InputPortWidth=4  IndexOptions=Index vector (dialog)  Indices=3  OutputSizes=1
  Selector3 : Selector  InputPortWidth=4  IndexOptions=Index vector (dialog)  Indices=4  OutputSizes=1
  Selector4 : Selector  InputPortWidth=4  IndexOptions=Index vector (dialog)  Indices=1  OutputSizes=1
  Selector5 : Selector  InputPortWidth=4  IndexOptions=Index vector (dialog)  Indices=1  OutputSizes=1
  Selector6 : Selector  InputPortWidth=4  IndexOptions=Index vector (dialog)  Indices=3  OutputSizes=1
  Selector7 : Selector  InputPortWidth=4  IndexOptions=Index vector (dialog)  Indices=3  OutputSizes=1
  Selector8 : Selector  InputPortWidth=4  IndexOptions=Index vector (dialog)  Indices=2  OutputSizes=1
  Selector9 : Selector  InputPortWidth=4  IndexOptions=Index vector (dialog)  Indices=2  OutputSizes=1
  Sensors : Subsystem
  Steering rack : Subsystem
      settings: PortSchema="{\"entries\":[{\"content\":{\"sides\":[{\"content\":{\"connectorIds\":[\"LConn1\"]},\"type\":\"ConnectorPlacement.EquallySpacedRectSide\",\"uuid\":\"6dc6ed4f-bc40-436b-b956-95802cd9ab0b\"},{\"content\":{\"connectorIds\":[\"RConn1\"],\"side\":\"RIGHT\"},\"type\":\"ConnectorPlacement.EquallySpacedRectSide\",\"uuid\":\"bd6c321d-8aef-4dea-b6e1-aa7a472e67f8\"},{\"content\":{\"connectorIds\":[],\"side\":\"TOP\"},\"type\":\"ConnectorPlacement.EquallySpacedRectSide\",\"uuid\":\"c6af7ff4-db9d-47e0-b1fc-962e1cf0b8c4\"},{\"content\":{\"connectorIds\":[\"RConn2\",\"In1\"],\"side\":\"BOTTOM\"},\"type\":\"ConnectorPlacement.EquallySpacedRectSide\",\"uuid\":\"93e0e125-4db7-45ac-b4d6-39baf46eca45\"}]},\"type\":\"ConnectorPlacement.EquallySpacedConnector\",\"uuid\":\"ca57ac6f-7feb-47cf-9a03-b9bce30e5561\"}],\"packageUris\":[\"http://schema.mathworks.com/mf0/Connector/19700101\"],\"version\":\"1.0\"}"
  Vehicle Motion : Subsystem
  Wheel - FL : tire/Wheel - Fiala  side=Left  sideSign=1  Brake_staticMu=Brakes_staticMu  Brake_kineticMu=Brakes_kineticMu  Brake_actuatorD=Brakes_caliperD  Brake_effectiveR=Brakes_effectiveR  Brake_pads=Brakes_pads_front  Tire_Cx=1e7  Tire_Cy=Tire_Cy  Tire_Cg=Tire_Cg  Tire_muKinetic=1.8  Tire_muStatic=2  Tire_Lx=Tire_Lx  Tire_Ly=Tire_Ly  Tire_radius=Tire_radius  Wheel_inertia=Wheel_inertia  Tire_Rdamp=0  Wheel_width=Wheel_width  Wheel_mass=Tire_mass + Rim_mass  Tire_prs=Tire_prs_front  Tire_Kz=Tire_VerticalK
  Wheel - FR : tire/Wheel - Fiala  side=Left  sideSign=1  Brake_staticMu=Brakes_staticMu  Brake_kineticMu=Brakes_kineticMu  Brake_actuatorD=Brakes_caliperD  Brake_effectiveR=Brakes_effectiveR  Brake_pads=Brakes_pads_front  Tire_Cx=1e7  Tire_Cy=Tire_Cy  Tire_Cg=Tire_Cg  Tire_muKinetic=1.8  Tire_muStatic=2  Tire_Lx=Tire_Lx  Tire_Ly=Tire_Ly  Tire_radius=Tire_radius  Wheel_inertia=Wheel_inertia  Tire_Rdamp=0  Wheel_width=Wheel_width  Wheel_mass=Tire_mass + Rim_mass  Tire_prs=Tire_prs_front  Tire_Kz=Tire_VerticalK
  Wheel - RL : tire/Wheel - Fiala  side=Left  sideSign=1  Brake_staticMu=Brakes_staticMu  Brake_kineticMu=Brakes_kineticMu  Brake_actuatorD=Brakes_caliperD  Brake_effectiveR=Brakes_effectiveR  Brake_pads=Brakes_pads_front  Tire_Cx=1e7  Tire_Cy=Tire_Cy  Tire_Cg=Tire_Cg  Tire_muKinetic=1.8  Tire_muStatic=2  Tire_Lx=Tire_Lx  Tire_Ly=Tire_Ly  Tire_radius=Tire_radius  Wheel_inertia=Wheel_inertia  Tire_Rdamp=0  Wheel_width=Wheel_width  Wheel_mass=Tire_mass + Rim_mass  Tire_prs=Tire_prs_front  Tire_Kz=Tire_VerticalK
  Wheel - RR : tire/Wheel - Fiala  side=Left  sideSign=1  Brake_staticMu=Brakes_staticMu  Brake_kineticMu=Brakes_kineticMu  Brake_actuatorD=Brakes_caliperD  Brake_effectiveR=Brakes_effectiveR  Brake_pads=Brakes_pads_front  Tire_Cx=1e7  Tire_Cy=Tire_Cy  Tire_Cg=Tire_Cg  Tire_muKinetic=1.8  Tire_muStatic=2  Tire_Lx=Tire_Lx  Tire_Ly=Tire_Ly  Tire_radius=Tire_radius  Wheel_inertia=Wheel_inertia  Tire_Rdamp=0  Wheel_width=Wheel_width  Wheel_mass=Tire_mass + Rim_mass  Tire_prs=Tire_prs_front  Tire_Kz=Tire_VerticalK
  GroundRef : PMIOPort  Side=Right
  Info : Outport
  tag scope: FL_TireInfo=global, FR_TireInfo=global, RL_TireInfo=global, RR_TireInfo=global
  wires:
  AxlTrq_3/1 -> Selector8/1
  Selector2/1 -> Wheel - RL/1
  Selector6/1 -> Wheel - RL/2
  GndZ_3/1 -> Selector7/1
  Selector7/1 -> Wheel - RL/3
  AxlTrq_2/1 -> Selector10/1
  Selector10/1 -> Wheel - RR/2
  GndZ_2/1 -> Selector11/1
  Rack ratio/1 -> Steering rack/1(Steering input)
  AxlTrq_1/1 -> Selector6/1
  BrkPrs/1 -> Selector/1
  Selector11/1 -> Wheel - RR/3
  AxlTrq/1 -> Selector4/1
  BrkPrs_2/1 -> Selector2/1
  GndZ_1/1 -> Selector9/1
  Selector9/1 -> Wheel - FR/3
  Selector/1 -> Wheel - FL/1
  Selector5/1 -> Wheel - FL/3
  Selector8/1 -> Wheel - FR/2
  Selector3/1 -> Wheel - RR/1
  GndZ/1 -> Selector5/1
  BrkPrs_1/1 -> Selector3/1
  Selector1/1 -> Wheel - FR/1
  BrkPrs_3/1 -> Selector1/1
  Selector4/1 -> Wheel - FL/2
  StrngAng/1 -> Rack ratio/1
  Wheel - FL/1 -> [FL_TireInfo]
  Wheel - RL/1 -> [RL_TireInfo]
  Wheel - FR/1 -> [FR_TireInfo]
  Wheel - RR/1 -> [RR_TireInfo]
  Sensors/1(Info) -> Info/1
  connections:
  Vehicle Motion/RConn1 -- Rear Right Suspension/LConn1 -- Rear Left Suspension/LConn1 -- ARB Rear/RConn2 -- Chassis & Aero/LConn1 -- Cameras/RConn1 -- Front Right Suspension/LConn3 -- ARB Front/RConn2 -- Front Left Suspension/LConn3 -- Steering rack/RConn2
  Rear Left Suspension/LConn2 -- ARB Rear/LConn1
  Front Left Suspension/LConn1 -- Steering rack/LConn1
  Wheel - RL/LConn1 -- Rear Left Suspension/RConn1
  Front Left Suspension/LConn2 -- ARB Front/LConn1
  Rear Right Suspension/LConn2 -- ARB Rear/RConn1
  Front Right Suspension/LConn2 -- ARB Front/RConn1
  Wheel - FR/LConn1 -- Front Right Suspension/RConn1
  Front Right Suspension/LConn1 -- Steering rack/RConn1
  Wheel - FL/RConn1 -- Connection Label2/LConn1
  Wheel - RR/LConn1 -- Rear Right Suspension/RConn1
  Wheel - FR/RConn1 -- Connection Label5/LConn1
  Front Left Suspension/RConn1 -- Wheel - FL/LConn1
  Wheel - RL/RConn1 -- Connection Label4/LConn1
  Wheel - RR/RConn1 -- Connection Label6/LConn1
  Connection Label/LConn1 -- GroundRef/RConn1

== system Full_Car/Wheels w sensor ==
  Bus Selector{1..4} (4 alike) : BusSelector  OutputSignals=Omega
  Transfer Fcn{1..4} (4 alike) : TransferFcn  Numerator=[10]  Denominator=[1 10]
  Vector Concatenate2 : Concatenate  NumInputs=4
  Out1 : Outport
  wires:
  [RL_TireInfo] -> Bus Selector3/1  "RL"
  [FL_TireInfo] -> Bus Selector1/1  "FL"
  Bus Selector1/1 -> Transfer Fcn1/1  "<Omega>"
  [RR_TireInfo] -> Bus Selector4/1  "RR"
  Bus Selector4/1 -> Transfer Fcn4/1  "<Omega>"
  Bus Selector3/1 -> Transfer Fcn3/1  "<Omega>"
  [FR_TireInfo] -> Bus Selector2/1  "FR"
  Bus Selector2/1 -> Transfer Fcn2/1  "<Omega>"
  Vector Concatenate2/1 -> Out1/1
  Transfer Fcn1/1 -> Vector Concatenate2/1
  Transfer Fcn2/1 -> Vector Concatenate2/2
  Transfer Fcn3/1 -> Vector Concatenate2/3
  Transfer Fcn4/1 -> Vector Concatenate2/4

== system Full_Car/Gearbox/Rotational friction ==
  note: Viscous friction
  note: Stribeck friction
  note: Coulomb friction
  inTrq : Inport
  w : Inport  Port=2
  Add : Sum  Inputs=+++
  Constant2 : Constant  Value=CoulombTrq
  Constant3 : Constant  Value=viscCoef
  Dead Zone{1..4} (4 alike) : DeadZone  LowerValue=-inf  UpperValue=0  SaturateOnIntegerOverflow=on
  Divide : Product  Inputs=*/
  Divide1 : Product  Inputs=*/
  Exp : Math  SaturateOnIntegerOverflow=on
  Gain : Gain  Gain=-1
  Product : Product  Inputs=3
  Product1 : Product
  Product2 : Product
  Square : Math  Operator=square  SaturateOnIntegerOverflow=on
  Stribeck peak : Constant  Value=(brkwyTrq-CoulombTrq)*sqrt(2*exp(1))
  Subtract : Sum  Inputs=+-
  Tanh : Trigonometry  Operator=tanh
  wCoulomb : Constant  Value=brkwyVel/10
  wStribeck : Constant  Value=brkwyVel*sqrt(2)
  outTrq : Outport
  wires:
  Subtract/1 -> Dead Zone1/1
  Stribeck peak/1 -> Product/1
  inTrq/1 -> Subtract/1
  wCoulomb/1 -> Divide1/2
  Constant2/1 -> Product1/1
  Tanh/1 -> Product1/2
  Product2/1 -> Dead Zone2/1  "visc"
  Gain/1 -> Exp/1
  Square/1 -> Gain/1
  Divide1/1 -> Tanh/1
  wStribeck/1 -> Divide/2
  Product/1 -> Dead Zone4/1  "strb"
  Constant3/1 -> Product2/2
  Product1/1 -> Dead Zone3/1  "coul"
  Divide/1 -> Square/1, Product/3
  Exp/1 -> Product/2
  w/1 -> Product2/1, Divide1/1, Divide/1
  Dead Zone1/1 -> outTrq/1
  Add/1 -> Subtract/2
  Dead Zone2/1 -> Add/1
  Dead Zone3/1 -> Add/2
  Dead Zone4/1 -> Add/3

== system Full_Car/GeoPosition/cart2Geo ==
  <pos> : Inport
  Add : Sum
  Add1 : Sum
  Constant4 : Constant  Value=R
  Constant5 : Constant  Value=refLat
  Constant6 : Constant  Value=R
  Constant7 : Constant  Value=refLon
  Cos : Trigonometry  Operator=cos
  Degrees to Radians : simulink_extras/Transformations/Degrees to Radians
  Divide : Product  Inputs=*/
  Divide1 : Product  Inputs=*/
  Product : Product
  Radians to Degrees : simulink_extras/Transformations/Radians to Degrees
  Radians to Degrees1 : simulink_extras/Transformations/Radians to Degrees
  Selector : Selector  InputPortWidth=3  IndexOptions=Index vector (dialog)  Indices=2  OutputSizes=1
  Selector1 : Selector  InputPortWidth=3  IndexOptions=Index vector (dialog)  Indices=1  OutputSizes=1
  Lat : Outport
  Lon : Outport  Port=2
  wires:
  Constant5/1 -> Add/2
  Constant7/1 -> Add1/2
  Constant6/1 -> Product/2
  Radians to Degrees1/1 -> Add1/1
  Degrees to Radians/1 -> Cos/1
  Product/1 -> Divide1/2
  Selector/1 -> Divide/1
  [lat] -> Degrees to Radians/1
  Divide1/1 -> Radians to Degrees1/1
  Selector1/1 -> Divide1/1
  <pos>/1 -> Selector1/1, Selector/1
  Add/1 -> [lat], Lat/1
  Add1/1 -> Lon/1
  Radians to Degrees/1 -> Add/1
  Divide/1 -> Radians to Degrees/1
  Constant4/1 -> Divide/2
  Cos/1 -> Product/1

== system Full_Car/Maniobras/Acc+Brk ==
  Demux : Demux  Tag=STV Demux  Outputs=3
      settings: DisplayOption=none
  FromWs : FromWorkspace  Tag=STV FromWs  VariableName=tuvar
      SigBuilderData=|
      | common.afterFinalStr = 'Extrapolate'
      | common.sampleTime = 0
      | dataSet.timeRange = [0 100]
      | dataSet.name = 'Input signals'
      | sbobj.Groups.Name = 'Input signals'
      | sbobj.Groups.Signals(1).Name = 'Brake pressure'
      | sbobj.Groups.Signals(1).XData = [0 7.9 7.9 10 10 92.3 92.3 93.5 93.5 100]
      | sbobj.Groups.Signals(1).YData = [0 0 1 1 0 0 1.01 1.01 0 0]
      | sbobj.Groups.Signals(2).Name = 'Motor torque'
      | sbobj.Groups.Signals(2).XData = [0 0.5 1.2 7 7 93.1 93.1 94.9 94.9 100]
      | sbobj.Groups.Signals(2).YData = [0 0 0.47 0.55 0 -0.00911651728553 1.2 1.2 -0.00934699103713 -0.01]
      | sbobj.Groups.Signals(3).Name = 'Steering angle'
      | sbobj.Groups.Signals(3).XData = [0 92.8 92.8 94.6 94.6 100]
      | sbobj.Groups.Signals(3).YData = [0 0 1.17 1.17 0 0]
      | sbobj.Groups.TimeRange = [0 100]
  Brake pressure : Outport  Tag=STV Outport
  Motor torque : Outport  Tag=STV Outport  Port=2
  Steering angle : Outport  Tag=STV Outport  Port=3
  wires:
  FromWs/1 -> Demux/1
  Demux/1 -> Brake pressure/1
  Demux/2 -> Motor torque/1
  Demux/3 -> Steering angle/1

== system Full_Car/Maniobras/Lat ==
  Demux : Demux  Tag=STV Demux  Outputs=3
      settings: DisplayOption=none
  FromWs : FromWorkspace  Tag=STV FromWs  VariableName=tuvar
      SigBuilderData=|
      | common.afterFinalStr = 'Extrapolate'
      | common.sampleTime = 0
      | dataSet.timeRange = [0 100]
      | dataSet.name = 'Input signals'
      | sbobj.Groups.Name = 'Input signals'
      | sbobj.Groups.Signals(1).Name = 'Brake pressure'
      | sbobj.Groups.Signals(1).XData = [0 92.3 92.3 93.5 93.5 100]
      | sbobj.Groups.Signals(1).YData = [0 0 1.21 1.21 0 0]
      | sbobj.Groups.Signals(2).Name = 'Motor torque'
      | sbobj.Groups.Signals(2).XData = [0 2.1 2.1 3.5 5 15 15 93.1 93.1 94.9 94.9 100]
      | sbobj.Groups.Signals(2).YData = [0.81 0.81 0.23 0.23 0.58 0.64 -0.000770550857284 -0.00911651728553 1.2 1.2 -0.00934699103713 -0.01]
      | sbobj.Groups.Signals(3).Name = 'Steering angle'
      | sbobj.Groups.Signals(3).XData = [0 2.5 4.2 6.5 20 20 92.8 92.8 94.6 94.6 100]
      | sbobj.Groups.Signals(3).YData = [0 0 0.11 0.15 0.15 0 0 1.17 1.17 0 0]
      | sbobj.Groups.TimeRange = [0 100]
  Brake pressure : Outport  Tag=STV Outport
  Motor torque : Outport  Tag=STV Outport  Port=2
  Steering angle : Outport  Tag=STV Outport  Port=3
  wires:
  FromWs/1 -> Demux/1
  Demux/1 -> Brake pressure/1
  Demux/2 -> Motor torque/1
  Demux/3 -> Steering angle/1

== system Full_Car/Maniobras/Lat - isoline ==
  Demux : Demux  Tag=STV Demux  Outputs=3
      settings: DisplayOption=none
  FromWs : FromWorkspace  Tag=STV FromWs  VariableName=tuvar
      SigBuilderData=|
      | common.afterFinalStr = 'Extrapolate'
      | common.sampleTime = 0
      | dataSet.timeRange = [0 100]
      | dataSet.name = 'Input signals'
      | sbobj.Groups.Name = 'Input signals'
      | sbobj.Groups.Signals(1).Name = 'Brake pressure'
      | sbobj.Groups.Signals(1).XData = [0 92.3 92.3 93.5 93.5 100]
      | sbobj.Groups.Signals(1).YData = [0 0 1.21 1.21 0 0]
      | sbobj.Groups.Signals(2).Name = 'Motor torque'
      | sbobj.Groups.Signals(2).XData = [0 1.1 2.7 5 7.6 8.7 20 20 93.1 93.1 94.9 94.9 100]
      | sbobj.Groups.Signals(2).YData = [0 0.34 0.46 0.15 0.1365 0.19 0.19 -0.000770550857284 -0.00911651728553 1.2 1.2 -0.00934699103713 -0.01]
      | sbobj.Groups.Signals(3).Name = 'Steering angle'
      | sbobj.Groups.Signals(3).XData = [0 3 3.2 3.5 4.1 4.3 4.58 7.5 8.7 20 20 87.6 87.6 92.8 92.8 94.6 94.6 100]
      | sbobj.Groups.Signals(3).YData = [0 0 0.3 0.3 -0.3 -0.3 0 0 -0.14 -0.14 0 0 -0.68 -0.68 1.17 1.17 0 0]
      | sbobj.Groups.TimeRange = [0 100]
  Brake pressure : Outport  Tag=STV Outport
  Motor torque : Outport  Tag=STV Outport  Port=2
  Steering angle : Outport  Tag=STV Outport  Port=3
  wires:
  FromWs/1 -> Demux/1
  Demux/1 -> Brake pressure/1
  Demux/2 -> Motor torque/1
  Demux/3 -> Steering angle/1
